simulink model "os_polyphase_synth_4096c"  (R2021a) (continued)
== system os_polyphase_synth_4096c/pfb_fir/pol1_in1_tap82 == (continued)
      | PCIN>>17+(A+D)*(A+D)+C+CARRYIN
      | PCIN>>17+(A+D)*(A+D)+CARRYIN
      | PCIN>>17+(A+D)*(A+D)+P
      | PCIN>>17+(A+D)*(A+D)+P+CARRYIN
      | PCIN>>17+(A+D)*B
      | PCIN>>17+(A+D)*B+C
      | PCIN>>17+(A+D)*B+C+CARRYIN
      | PCIN>>17+(A+D)*B+CARRYIN
      | PCIN>>17+(A+D)*B+P
      | PCIN>>17+(A+D)*B+P+CARRYIN
      | PCIN>>17+(A+D)*BCIN
      | PCIN>>17+(A+D)*BCIN+C
      | PCIN>>17+(A+D)*BCIN+C+CARRYIN
      | PCIN>>17+(A+D)*BCIN+CARRYIN
      | PCIN>>17+(A+D)*BCIN+P
      | PCIN>>17+(A+D)*BCIN+P+CARRYIN
      | PCIN>>17+(A+D)+C
      | PCIN>>17+(A+D)+C+CARRYIN
      | PCIN>>17+(A+D)+CARRYIN
      | PCIN>>17+(A+D)+P
      | PCIN>>17+(A+D)+P+CARRYIN
      | PCIN>>17+(ACIN+D)
      | PCIN>>17+(ACIN+D)*(ACIN+D)
      | PCIN>>17+(ACIN+D)*(ACIN+D)+C
      | PCIN>>17+(ACIN+D)*(ACIN+D)+C+CARRYIN
      | PCIN>>17+(ACIN+D)*(ACIN+D)+CARRYIN
      | PCIN>>17+(ACIN+D)*(ACIN+D)+P
      | PCIN>>17+(ACIN+D)*(ACIN+D)+P+CARRYIN
      | PCIN>>17+(ACIN+D)*B
      | PCIN>>17+(ACIN+D)*B+C
      | PCIN>>17+(ACIN+D)*B+C+CARRYIN
      | PCIN>>17+(ACIN+D)*B+CARRYIN
      | PCIN>>17+(ACIN+D)*B+P
      | PCIN>>17+(ACIN+D)*B+P+CARRYIN
      | PCIN>>17+(ACIN+D)*BCIN
      | PCIN>>17+(ACIN+D)*BCIN+C
      | PCIN>>17+(ACIN+D)*BCIN+C+CARRYIN
      | PCIN>>17+(ACIN+D)*BCIN+CARRYIN
      | PCIN>>17+(ACIN+D)*BCIN+P
      | PCIN>>17+(ACIN+D)*BCIN+P+CARRYIN
      | PCIN>>17+(ACIN+D)+C
      | PCIN>>17+(ACIN+D)+C+CARRYIN
      | PCIN>>17+(ACIN+D)+CARRYIN
      | PCIN>>17+(ACIN+D)+P
      | PCIN>>17+(ACIN+D)+P+CARRYIN
      | PCIN>>17+(D+A)
      | PCIN>>17+(D+A)*(D+A)
      | PCIN>>17+(D+A)*(D+A)+C
      | PCIN>>17+(D+A)*(D+A)+C+CARRYIN
      | PCIN>>17+(D+A)*(D+A)+CARRYIN
      | PCIN>>17+(D+A)*(D+A)+P
      | PCIN>>17+(D+A)*(D+A)+P+CARRYIN
      | PCIN>>17+(D+A)*B
      | PCIN>>17+(D+A)*B+C
      | PCIN>>17+(D+A)*B+C+CARRYIN
      | PCIN>>17+(D+A)*B+CARRYIN
      | PCIN>>17+(D+A)*B+P
      | PCIN>>17+(D+A)*B+P+CARRYIN
      | PCIN>>17+(D+A)*BCIN
      | PCIN>>17+(D+A)*BCIN+C
      | PCIN>>17+(D+A)*BCIN+C+CARRYIN
      | PCIN>>17+(D+A)*BCIN+CARRYIN
      | PCIN>>17+(D+A)*BCIN+P
      | PCIN>>17+(D+A)*BCIN+P+CARRYIN
      | PCIN>>17+(D+A)+C
      | PCIN>>17+(D+A)+C+CARRYIN
      | PCIN>>17+(D+A)+CARRYIN
      | PCIN>>17+(D+A)+P
      | PCIN>>17+(D+A)+P+CARRYIN
      | PCIN>>17+(D+ACIN)
      | PCIN>>17+(D+ACIN)*(D+ACIN)
      | PCIN>>17+(D+ACIN)*(D+ACIN)+C
      | PCIN>>17+(D+ACIN)*(D+ACIN)+C+CARRYIN
      | PCIN>>17+(D+ACIN)*(D+ACIN)+CARRYIN
      | PCIN>>17+(D+ACIN)*(D+ACIN)+P
      | PCIN>>17+(D+ACIN)*(D+ACIN)+P+CARRYIN
      | PCIN>>17+(D+ACIN)*B
      | PCIN>>17+(D+ACIN)*B+C
      | PCIN>>17+(D+ACIN)*B+C+CARRYIN
      | PCIN>>17+(D+ACIN)*B+CARRYIN
      | PCIN>>17+(D+ACIN)*B+P
      | PCIN>>17+(D+ACIN)*B+P+CARRYIN
      | PCIN>>17+(D+ACIN)*BCIN
      | PCIN>>17+(D+ACIN)*BCIN+C
      | PCIN>>17+(D+ACIN)*BCIN+C+CARRYIN
      | PCIN>>17+(D+ACIN)*BCIN+CARRYIN
      | PCIN>>17+(D+ACIN)*BCIN+P
      | PCIN>>17+(D+ACIN)*BCIN+P+CARRYIN
      | PCIN>>17+(D+ACIN)+C
      | PCIN>>17+(D+ACIN)+C+CARRYIN
      | PCIN>>17+(D+ACIN)+CARRYIN
      | PCIN>>17+(D+ACIN)+P
      | PCIN>>17+(D+ACIN)+P+CARRYIN
      | PCIN>>17+(D-A)
      | PCIN>>17+(D-A)*(D-A)
      | PCIN>>17+(D-A)*(D-A)+C
      | PCIN>>17+(D-A)*(D-A)+C+CARRYIN
      | PCIN>>17+(D-A)*(D-A)+CARRYIN
      | PCIN>>17+(D-A)*(D-A)+P
      | PCIN>>17+(D-A)*(D-A)+P+CARRYIN
      | PCIN>>17+(D-A)*B
      | PCIN>>17+(D-A)*B+C
      | PCIN>>17+(D-A)*B+C+CARRYIN
      | PCIN>>17+(D-A)*B+CARRYIN
      | PCIN>>17+(D-A)*B+P
      | PCIN>>17+(D-A)*B+P+CARRYIN
      | PCIN>>17+(D-A)*BCIN
      | PCIN>>17+(D-A)*BCIN+C
      | PCIN>>17+(D-A)*BCIN+C+CARRYIN
      | PCIN>>17+(D-A)*BCIN+CARRYIN
      | PCIN>>17+(D-A)*BCIN+P
      | PCIN>>17+(D-A)*BCIN+P+CARRYIN
      | PCIN>>17+(D-A)+C
      | PCIN>>17+(D-A)+C+CARRYIN
      | PCIN>>17+(D-A)+CARRYIN
      | PCIN>>17+(D-A)+P
      | PCIN>>17+(D-A)+P+CARRYIN
      | PCIN>>17+(D-ACIN)
      | PCIN>>17+(D-ACIN)*(D-ACIN)
      | PCIN>>17+(D-ACIN)*(D-ACIN)+C
      | PCIN>>17+(D-ACIN)*(D-ACIN)+C+CARRYIN
      | PCIN>>17+(D-ACIN)*(D-ACIN)+CARRYIN
      | PCIN>>17+(D-ACIN)*(D-ACIN)+P
      | PCIN>>17+(D-ACIN)*(D-ACIN)+P+CARRYIN
      | PCIN>>17+(D-ACIN)*B
      | PCIN>>17+(D-ACIN)*B+C
      | PCIN>>17+(D-ACIN)*B+C+CARRYIN
      | PCIN>>17+(D-ACIN)*B+CARRYIN
      | PCIN>>17+(D-ACIN)*B+P
      | PCIN>>17+(D-ACIN)*B+P+CARRYIN
      | PCIN>>17+(D-ACIN)*BCIN
      | PCIN>>17+(D-ACIN)*BCIN+C
      | PCIN>>17+(D-ACIN)*BCIN+C+CARRYIN
      | PCIN>>17+(D-ACIN)*BCIN+CARRYIN
      | PCIN>>17+(D-ACIN)*BCIN+P
      | PCIN>>17+(D-ACIN)*BCIN+P+CARRYIN
      | PCIN>>17+(D-ACIN)+C
      | PCIN>>17+(D-ACIN)+C+CARRYIN
      | PCIN>>17+(D-ACIN)+CARRYIN
      | PCIN>>17+(D-ACIN)+P
      | PCIN>>17+(D-ACIN)+P+CARRYIN
      | PCIN>>17+A
      | PCIN>>17+A*A
      | PCIN>>17+A*A+C
      | PCIN>>17+A*A+C+CARRYIN
      | PCIN>>17+A*A+CARRYIN
      | PCIN>>17+A*A+P
      | PCIN>>17+A*A+P+CARRYIN
      | PCIN>>17+A*B
      | PCIN>>17+A*B+C
      | PCIN>>17+A*B+C+CARRYIN
      | PCIN>>17+A*B+CARRYIN
      | PCIN>>17+A*B+P
      | PCIN>>17+A*B+P+CARRYIN
      | PCIN>>17+A*BCIN
      | PCIN>>17+A*BCIN+C
      | PCIN>>17+A*BCIN+C+CARRYIN
      | PCIN>>17+A*BCIN+CARRYIN
      | PCIN>>17+A*BCIN+P
      | PCIN>>17+A*BCIN+P+CARRYIN
      | PCIN>>17+A+C
      | PCIN>>17+A+C+CARRYIN
      | PCIN>>17+A+CARRYIN
      | PCIN>>17+A+P
      | PCIN>>17+A+P+CARRYIN
      | PCIN>>17+ACIN
      | PCIN>>17+ACIN*ACIN
      | PCIN>>17+ACIN*ACIN+C
      | PCIN>>17+ACIN*ACIN+C+CARRYIN
      | PCIN>>17+ACIN*ACIN+CARRYIN
      | PCIN>>17+ACIN*ACIN+P
      | PCIN>>17+ACIN*ACIN+P+CARRYIN
      | PCIN>>17+ACIN*B
      | PCIN>>17+ACIN*B+C
      | PCIN>>17+ACIN*B+C+CARRYIN
      | PCIN>>17+ACIN*B+CARRYIN
      | PCIN>>17+ACIN*B+P
      | PCIN>>17+ACIN*B+P+CARRYIN
      | PCIN>>17+ACIN*BCIN
      | PCIN>>17+ACIN*BCIN+C
      | PCIN>>17+ACIN*BCIN+C+CARRYIN
      | PCIN>>17+ACIN*BCIN+CARRYIN
      | PCIN>>17+ACIN*BCIN+P
      | PCIN>>17+ACIN*BCIN+P+CARRYIN
      | PCIN>>17+ACIN+C
      | PCIN>>17+ACIN+C+CARRYIN
      | PCIN>>17+ACIN+CARRYIN
      | PCIN>>17+ACIN+P
      | PCIN>>17+ACIN+P+CARRYIN
      | PCIN>>17+B*(A+D)
      | PCIN>>17+B*(A+D)+C
      | PCIN>>17+B*(A+D)+C+CARRYIN
      | PCIN>>17+B*(A+D)+CARRYIN
      | PCIN>>17+B*(A+D)+P
      | PCIN>>17+B*(A+D)+P+CARRYIN
      | PCIN>>17+B*(ACIN+D)
      | PCIN>>17+B*(ACIN+D)+C
      | PCIN>>17+B*(ACIN+D)+C+CARRYIN
      | PCIN>>17+B*(ACIN+D)+CARRYIN
      | PCIN>>17+B*(ACIN+D)+P
      | PCIN>>17+B*(ACIN+D)+P+CARRYIN
      | PCIN>>17+B*(D+A)
      | PCIN>>17+B*(D+A)+C
      | PCIN>>17+B*(D+A)+C+CARRYIN
      | PCIN>>17+B*(D+A)+CARRYIN
      | PCIN>>17+B*(D+A)+P
      | PCIN>>17+B*(D+A)+P+CARRYIN
      | PCIN>>17+B*(D+ACIN)
      | PCIN>>17+B*(D+ACIN)+C
      | PCIN>>17+B*(D+ACIN)+C+CARRYIN
      | PCIN>>17+B*(D+ACIN)+CARRYIN
      | PCIN>>17+B*(D+ACIN)+P
      | PCIN>>17+B*(D+ACIN)+P+CARRYIN
      | PCIN>>17+B*(D-A)
      | PCIN>>17+B*(D-A)+C
      | PCIN>>17+B*(D-A)+C+CARRYIN
      | PCIN>>17+B*(D-A)+CARRYIN
      | PCIN>>17+B*(D-A)+P
      | PCIN>>17+B*(D-A)+P+CARRYIN
      | PCIN>>17+B*(D-ACIN)
      | PCIN>>17+B*(D-ACIN)+C
      | PCIN>>17+B*(D-ACIN)+C+CARRYIN
      | PCIN>>17+B*(D-ACIN)+CARRYIN
      | PCIN>>17+B*(D-ACIN)+P
      | PCIN>>17+B*(D-ACIN)+P+CARRYIN
      | PCIN>>17+B*A
      | PCIN>>17+B*A+C
      | PCIN>>17+B*A+C+CARRYIN
      | PCIN>>17+B*A+CARRYIN
      | PCIN>>17+B*A+P
      | PCIN>>17+B*A+P+CARRYIN
      | PCIN>>17+B*ACIN
      | PCIN>>17+B*ACIN+C
      | PCIN>>17+B*ACIN+C+CARRYIN
      | PCIN>>17+B*ACIN+CARRYIN
      | PCIN>>17+B*ACIN+P
      | PCIN>>17+B*ACIN+P+CARRYIN
      | PCIN>>17+B*D
      | PCIN>>17+B*D+C
      | PCIN>>17+B*D+C+CARRYIN
      | PCIN>>17+B*D+CARRYIN
      | PCIN>>17+B*D+P
      | PCIN>>17+B*D+P+CARRYIN
      | PCIN>>17+BCIN*(A+D)
      | PCIN>>17+BCIN*(A+D)+C
      | PCIN>>17+BCIN*(A+D)+C+CARRYIN
      | PCIN>>17+BCIN*(A+D)+CARRYIN
      | PCIN>>17+BCIN*(A+D)+P
      | PCIN>>17+BCIN*(A+D)+P+CARRYIN
      | PCIN>>17+BCIN*(ACIN+D)
      | PCIN>>17+BCIN*(ACIN+D)+C
      | PCIN>>17+BCIN*(ACIN+D)+C+CARRYIN
      | PCIN>>17+BCIN*(ACIN+D)+CARRYIN
      | PCIN>>17+BCIN*(ACIN+D)+P
      | PCIN>>17+BCIN*(ACIN+D)+P+CARRYIN
      | PCIN>>17+BCIN*(D+A)
      | PCIN>>17+BCIN*(D+A)+C
      | PCIN>>17+BCIN*(D+A)+C+CARRYIN
      | PCIN>>17+BCIN*(D+A)+CARRYIN
      | PCIN>>17+BCIN*(D+A)+P
      | PCIN>>17+BCIN*(D+A)+P+CARRYIN
      | PCIN>>17+BCIN*(D+ACIN)
      | PCIN>>17+BCIN*(D+ACIN)+C
      | PCIN>>17+BCIN*(D+ACIN)+C+CARRYIN
      | PCIN>>17+BCIN*(D+ACIN)+CARRYIN
      | PCIN>>17+BCIN*(D+ACIN)+P
      | PCIN>>17+BCIN*(D+ACIN)+P+CARRYIN
      | PCIN>>17+BCIN*(D-A)
      | PCIN>>17+BCIN*(D-A)+C
      | PCIN>>17+BCIN*(D-A)+C+CARRYIN
      | PCIN>>17+BCIN*(D-A)+CARRYIN
      | PCIN>>17+BCIN*(D-A)+P
      | PCIN>>17+BCIN*(D-A)+P+CARRYIN
      | PCIN>>17+BCIN*(D-ACIN)
      | PCIN>>17+BCIN*(D-ACIN)+C
      | PCIN>>17+BCIN*(D-ACIN)+C+CARRYIN
      | PCIN>>17+BCIN*(D-ACIN)+CARRYIN
      | PCIN>>17+BCIN*(D-ACIN)+P
      | PCIN>>17+BCIN*(D-ACIN)+P+CARRYIN
      | PCIN>>17+BCIN*A
      | PCIN>>17+BCIN*A+C
      | PCIN>>17+BCIN*A+C+CARRYIN
      | PCIN>>17+BCIN*A+CARRYIN
      | PCIN>>17+BCIN*A+P
      | PCIN>>17+BCIN*A+P+CARRYIN
      | PCIN>>17+BCIN*ACIN
      | PCIN>>17+BCIN*ACIN+C
      | PCIN>>17+BCIN*ACIN+C+CARRYIN
      | PCIN>>17+BCIN*ACIN+CARRYIN
      | PCIN>>17+BCIN*ACIN+P
      | PCIN>>17+BCIN*ACIN+P+CARRYIN
      | PCIN>>17+BCIN*D
      | PCIN>>17+BCIN*D+C
      | PCIN>>17+BCIN*D+C+CARRYIN
      | PCIN>>17+BCIN*D+CARRYIN
      | PCIN>>17+BCIN*D+P
      | PCIN>>17+BCIN*D+P+CARRYIN
      | PCIN>>17+C
      | PCIN>>17+C+(A+D)
      | PCIN>>17+C+(A+D)*(A+D)
      | PCIN>>17+C+(A+D)*(A+D)+CARRYIN
      | PCIN>>17+C+(A+D)*B
      | PCIN>>17+C+(A+D)*B+CARRYIN
      | PCIN>>17+C+(A+D)*BCIN
      | PCIN>>17+C+(A+D)*BCIN+CARRYIN
      | PCIN>>17+C+(A+D)+CARRYIN
      | PCIN>>17+C+(ACIN+D)
      | PCIN>>17+C+(ACIN+D)*(ACIN+D)
      | PCIN>>17+C+(ACIN+D)*(ACIN+D)+CARRYIN
      | PCIN>>17+C+(ACIN+D)*B
      | PCIN>>17+C+(ACIN+D)*B+CARRYIN
      | PCIN>>17+C+(ACIN+D)*BCIN
      | PCIN>>17+C+(ACIN+D)*BCIN+CARRYIN
      | PCIN>>17+C+(ACIN+D)+CARRYIN
      | PCIN>>17+C+(D+A)
      | PCIN>>17+C+(D+A)*(D+A)
      | PCIN>>17+C+(D+A)*(D+A)+CARRYIN
      | PCIN>>17+C+(D+A)*B
      | PCIN>>17+C+(D+A)*B+CARRYIN
      | PCIN>>17+C+(D+A)*BCIN
      | PCIN>>17+C+(D+A)*BCIN+CARRYIN
      | PCIN>>17+C+(D+A)+CARRYIN
      | PCIN>>17+C+(D+ACIN)
      | PCIN>>17+C+(D+ACIN)*(D+ACIN)
      | PCIN>>17+C+(D+ACIN)*(D+ACIN)+CARRYIN
      | PCIN>>17+C+(D+ACIN)*B
      | PCIN>>17+C+(D+ACIN)*B+CARRYIN
      | PCIN>>17+C+(D+ACIN)*BCIN
      | PCIN>>17+C+(D+ACIN)*BCIN+CARRYIN
      | PCIN>>17+C+(D+ACIN)+CARRYIN
      | PCIN>>17+C+(D-A)
      | PCIN>>17+C+(D-A)*(D-A)
      | PCIN>>17+C+(D-A)*(D-A)+CARRYIN
      | PCIN>>17+C+(D-A)*B
      | PCIN>>17+C+(D-A)*B+CARRYIN
      | PCIN>>17+C+(D-A)*BCIN
      | PCIN>>17+C+(D-A)*BCIN+CARRYIN
      | PCIN>>17+C+(D-A)+CARRYIN
      | PCIN>>17+C+(D-ACIN)
      | PCIN>>17+C+(D-ACIN)*(D-ACIN)
      | PCIN>>17+C+(D-ACIN)*(D-ACIN)+CARRYIN
      | PCIN>>17+C+(D-ACIN)*B
      | PCIN>>17+C+(D-ACIN)*B+CARRYIN
      | PCIN>>17+C+(D-ACIN)*BCIN
      | PCIN>>17+C+(D-ACIN)*BCIN+CARRYIN
      | PCIN>>17+C+(D-ACIN)+CARRYIN
      | PCIN>>17+C+A
      | PCIN>>17+C+A*A
      | PCIN>>17+C+A*A+CARRYIN
      | PCIN>>17+C+A*B
      | PCIN>>17+C+A*B+CARRYIN
      | PCIN>>17+C+A*BCIN
      | PCIN>>17+C+A*BCIN+CARRYIN
      | PCIN>>17+C+A+CARRYIN
      | PCIN>>17+C+ACIN
      | PCIN>>17+C+ACIN*ACIN
      | PCIN>>17+C+ACIN*ACIN+CARRYIN
      | PCIN>>17+C+ACIN*B
      | PCIN>>17+C+ACIN*B+CARRYIN
      | PCIN>>17+C+ACIN*BCIN
      | PCIN>>17+C+ACIN*BCIN+CARRYIN
      | PCIN>>17+C+ACIN+CARRYIN
      | PCIN>>17+C+B*(A+D)
      | PCIN>>17+C+B*(A+D)+CARRYIN
      | PCIN>>17+C+B*(ACIN+D)
      | PCIN>>17+C+B*(ACIN+D)+CARRYIN
      | PCIN>>17+C+B*(D+A)
      | PCIN>>17+C+B*(D+A)+CARRYIN
      | PCIN>>17+C+B*(D+ACIN)
      | PCIN>>17+C+B*(D+ACIN)+CARRYIN
      | PCIN>>17+C+B*(D-A)
      | PCIN>>17+C+B*(D-A)+CARRYIN
      | PCIN>>17+C+B*(D-ACIN)
      | PCIN>>17+C+B*(D-ACIN)+CARRYIN
      | PCIN>>17+C+B*A
      | PCIN>>17+C+B*A+CARRYIN
      | PCIN>>17+C+B*ACIN
      | PCIN>>17+C+B*ACIN+CARRYIN
      | PCIN>>17+C+B*D
      | PCIN>>17+C+B*D+CARRYIN
      | PCIN>>17+C+BCIN*(A+D)
      | PCIN>>17+C+BCIN*(A+D)+CARRYIN
      | PCIN>>17+C+BCIN*(ACIN+D)
      | PCIN>>17+C+BCIN*(ACIN+D)+CARRYIN
      | PCIN>>17+C+BCIN*(D+A)
      | PCIN>>17+C+BCIN*(D+A)+CARRYIN
      | PCIN>>17+C+BCIN*(D+ACIN)
      | PCIN>>17+C+BCIN*(D+ACIN)+CARRYIN
      | PCIN>>17+C+BCIN*(D-A)
      | PCIN>>17+C+BCIN*(D-A)+CARRYIN
      | PCIN>>17+C+BCIN*(D-ACIN)
      | PCIN>>17+C+BCIN*(D-ACIN)+CARRYIN
      | PCIN>>17+C+BCIN*A
      | PCIN>>17+C+BCIN*A+CARRYIN
      | PCIN>>17+C+BCIN*ACIN
      | PCIN>>17+C+BCIN*ACIN+CARRYIN
      | PCIN>>17+C+BCIN*D
      | PCIN>>17+C+BCIN*D+CARRYIN
      | PCIN>>17+C+C
      | PCIN>>17+C+C+CARRYIN
      | PCIN>>17+C+C+CONCAT
      | PCIN>>17+C+C+CONCAT+CARRYIN
      | PCIN>>17+C+C+P
      | PCIN>>17+C+C+P+CARRYIN
      | PCIN>>17+C+CARRYIN
      | PCIN>>17+C+CONCAT
      | PCIN>>17+C+CONCAT+C
      | PCIN>>17+C+CONCAT+C+CARRYIN
      | PCIN>>17+C+CONCAT+CARRYIN
      | PCIN>>17+C+CONCAT+P
      | PCIN>>17+C+CONCAT+P+CARRYIN
      | PCIN>>17+C+D
      | PCIN>>17+C+D*B
      | PCIN>>17+C+D*B+CARRYIN
      | PCIN>>17+C+D*BCIN
      | PCIN>>17+C+D*BCIN+CARRYIN
      | PCIN>>17+C+D*D
      | PCIN>>17+C+D*D+CARRYIN
      | PCIN>>17+C+D+CARRYIN
      | PCIN>>17+C+P
      | PCIN>>17+C+P+C
      | PCIN>>17+C+P+C+CARRYIN
      | PCIN>>17+C+P+CARRYIN
      | PCIN>>17+C+P+CONCAT
      | PCIN>>17+C+P+CONCAT+CARRYIN
      | PCIN>>17+C+P+P
      | PCIN>>17+C+P+P+CARRYIN
      | PCIN>>17+CARRYIN
      | PCIN>>17+CONCAT
      | PCIN>>17+CONCAT+C
      | PCIN>>17+CONCAT+C+C
      | PCIN>>17+CONCAT+C+C+CARRYIN
      | PCIN>>17+CONCAT+C+CARRYIN
      | PCIN>>17+CONCAT+C+P
      | PCIN>>17+CONCAT+C+P+CARRYIN
      | PCIN>>17+CONCAT+CARRYIN
      | PCIN>>17+CONCAT+P
      | PCIN>>17+CONCAT+P+C
      | PCIN>>17+CONCAT+P+C+CARRYIN
      | PCIN>>17+CONCAT+P+CARRYIN
      | PCIN>>17+D
      | PCIN>>17+D*B
      | PCIN>>17+D*B+C
      | PCIN>>17+D*B+C+CARRYIN
      | PCIN>>17+D*B+CARRYIN
      | PCIN>>17+D*B+P
      | PCIN>>17+D*B+P+CARRYIN
      | PCIN>>17+D*BCIN
      | PCIN>>17+D*BCIN+C
      | PCIN>>17+D*BCIN+C+CARRYIN
      | PCIN>>17+D*BCIN+CARRYIN
      | PCIN>>17+D*BCIN+P
      | PCIN>>17+D*BCIN+P+CARRYIN
      | PCIN>>17+D*D
      | PCIN>>17+D*D+C
      | PCIN>>17+D*D+C+CARRYIN
      | PCIN>>17+D*D+CARRYIN
      | PCIN>>17+D*D+P
      | PCIN>>17+D*D+P+CARRYIN
      | PCIN>>17+D+C
      | PCIN>>17+D+C+CARRYIN
      | PCIN>>17+D+CARRYIN
      | PCIN>>17+D+P
      | PCIN>>17+D+P+CARRYIN
      | PCIN>>17+P
      | PCIN>>17+P+(A+D)
      | PCIN>>17+P+(A+D)*(A+D)
      | PCIN>>17+P+(A+D)*(A+D)+CARRYIN
      | PCIN>>17+P+(A+D)*B
      | PCIN>>17+P+(A+D)*B+CARRYIN
      | PCIN>>17+P+(A+D)*BCIN
      | PCIN>>17+P+(A+D)*BCIN+CARRYIN
      | PCIN>>17+P+(A+D)+CARRYIN
      | PCIN>>17+P+(ACIN+D)
      | PCIN>>17+P+(ACIN+D)*(ACIN+D)
      | PCIN>>17+P+(ACIN+D)*(ACIN+D)+CARRYIN
      | PCIN>>17+P+(ACIN+D)*B
      | PCIN>>17+P+(ACIN+D)*B+CARRYIN
      | PCIN>>17+P+(ACIN+D)*BCIN
      | PCIN>>17+P+(ACIN+D)*BCIN+CARRYIN
      | PCIN>>17+P+(ACIN+D)+CARRYIN
      | PCIN>>17+P+(D+A)
      | PCIN>>17+P+(D+A)*(D+A)
      | PCIN>>17+P+(D+A)*(D+A)+CARRYIN
      | PCIN>>17+P+(D+A)*B
      | PCIN>>17+P+(D+A)*B+CARRYIN
      | PCIN>>17+P+(D+A)*BCIN
      | PCIN>>17+P+(D+A)*BCIN+CARRYIN
      | PCIN>>17+P+(D+A)+CARRYIN
      | PCIN>>17+P+(D+ACIN)
      | PCIN>>17+P+(D+ACIN)*(D+ACIN)
      | PCIN>>17+P+(D+ACIN)*(D+ACIN)+CARRYIN
      | PCIN>>17+P+(D+ACIN)*B
      | PCIN>>17+P+(D+ACIN)*B+CARRYIN
      | PCIN>>17+P+(D+ACIN)*BCIN
      | PCIN>>17+P+(D+ACIN)*BCIN+CARRYIN
      | PCIN>>17+P+(D+ACIN)+CARRYIN
      | PCIN>>17+P+(D-A)
      | PCIN>>17+P+(D-A)*(D-A)
      | PCIN>>17+P+(D-A)*(D-A)+CARRYIN
      | PCIN>>17+P+(D-A)*B
      | PCIN>>17+P+(D-A)*B+CARRYIN
      | PCIN>>17+P+(D-A)*BCIN
      | PCIN>>17+P+(D-A)*BCIN+CARRYIN
      | PCIN>>17+P+(D-A)+CARRYIN
      | PCIN>>17+P+(D-ACIN)
      | PCIN>>17+P+(D-ACIN)*(D-ACIN)
      | PCIN>>17+P+(D-ACIN)*(D-ACIN)+CARRYIN
      | PCIN>>17+P+(D-ACIN)*B
      | PCIN>>17+P+(D-ACIN)*B+CARRYIN
      | PCIN>>17+P+(D-ACIN)*BCIN
      | PCIN>>17+P+(D-ACIN)*BCIN+CARRYIN
      | PCIN>>17+P+(D-ACIN)+CARRYIN
      | PCIN>>17+P+A
      | PCIN>>17+P+A*A
      | PCIN>>17+P+A*A+CARRYIN
      | PCIN>>17+P+A*B
      | PCIN>>17+P+A*B+CARRYIN
      | PCIN>>17+P+A*BCIN
      | PCIN>>17+P+A*BCIN+CARRYIN
      | PCIN>>17+P+A+CARRYIN
      | PCIN>>17+P+ACIN
      | PCIN>>17+P+ACIN*ACIN
      | PCIN>>17+P+ACIN*ACIN+CARRYIN
      | PCIN>>17+P+ACIN*B
      | PCIN>>17+P+ACIN*B+CARRYIN
      | PCIN>>17+P+ACIN*BCIN
      | PCIN>>17+P+ACIN*BCIN+CARRYIN
      | PCIN>>17+P+ACIN+CARRYIN
      | PCIN>>17+P+B*(A+D)
      | PCIN>>17+P+B*(A+D)+CARRYIN
      | PCIN>>17+P+B*(ACIN+D)
      | PCIN>>17+P+B*(ACIN+D)+CARRYIN
      | PCIN>>17+P+B*(D+A)
      | PCIN>>17+P+B*(D+A)+CARRYIN
      | PCIN>>17+P+B*(D+ACIN)
      | PCIN>>17+P+B*(D+ACIN)+CARRYIN
      | PCIN>>17+P+B*(D-A)
      | PCIN>>17+P+B*(D-A)+CARRYIN
      | PCIN>>17+P+B*(D-ACIN)
      | PCIN>>17+P+B*(D-ACIN)+CARRYIN
      | PCIN>>17+P+B*A
      | PCIN>>17+P+B*A+CARRYIN
      | PCIN>>17+P+B*ACIN
      | PCIN>>17+P+B*ACIN+CARRYIN
      | PCIN>>17+P+B*D
      | PCIN>>17+P+B*D+CARRYIN
      | PCIN>>17+P+BCIN*(A+D)
      | PCIN>>17+P+BCIN*(A+D)+CARRYIN
      | PCIN>>17+P+BCIN*(ACIN+D)
      | PCIN>>17+P+BCIN*(ACIN+D)+CARRYIN
      | PCIN>>17+P+BCIN*(D+A)
      | PCIN>>17+P+BCIN*(D+A)+CARRYIN
      | PCIN>>17+P+BCIN*(D+ACIN)
      | PCIN>>17+P+BCIN*(D+ACIN)+CARRYIN
      | PCIN>>17+P+BCIN*(D-A)
      | PCIN>>17+P+BCIN*(D-A)+CARRYIN
      | PCIN>>17+P+BCIN*(D-ACIN)
      | PCIN>>17+P+BCIN*(D-ACIN)+CARRYIN
      | PCIN>>17+P+BCIN*A
      | PCIN>>17+P+BCIN*A+CARRYIN
      | PCIN>>17+P+BCIN*ACIN
      | PCIN>>17+P+BCIN*ACIN+CARRYIN
      | PCIN>>17+P+BCIN*D
      | PCIN>>17+P+BCIN*D+CARRYIN
      | PCIN>>17+P+C
      | PCIN>>17+P+C+C
      | PCIN>>17+P+C+C+CARRYIN
      | PCIN>>17+P+C+CARRYIN
      | PCIN>>17+P+C+CONCAT
      | PCIN>>17+P+C+CONCAT+CARRYIN
      | PCIN>>17+P+C+P
      | PCIN>>17+P+C+P+CARRYIN
      | PCIN>>17+P+CARRYIN
      | PCIN>>17+P+CONCAT
      | PCIN>>17+P+CONCAT+C
      | PCIN>>17+P+CONCAT+C+CARRYIN
      | PCIN>>17+P+CONCAT+CARRYIN
      | PCIN>>17+P+D
      | PCIN>>17+P+D*B
      | PCIN>>17+P+D*B+CARRYIN
      | PCIN>>17+P+D*BCIN
      | PCIN>>17+P+D*BCIN+CARRYIN
      | PCIN>>17+P+D*D
      | PCIN>>17+P+D*D+CARRYIN
      | PCIN>>17+P+D+CARRYIN
      | PCIN>>17+P+P
      | PCIN>>17+P+P+C
      | PCIN>>17+P+P+C+CARRYIN
      | PCIN>>17+P+P+CARRYIN
      | PCIN>>17-((A+D))
      | PCIN>>17-((A+D)*(A+D))
      | PCIN>>17-((A+D)*(A+D)+C)
      | PCIN>>17-((A+D)*(A+D)+C+CARRYIN)
      | PCIN>>17-((A+D)*(A+D)+CARRYIN)
      | PCIN>>17-((A+D)*(A+D)+P)
      | PCIN>>17-((A+D)*(A+D)+P+CARRYIN)
      | PCIN>>17-((A+D)*B)
      | PCIN>>17-((A+D)*B+C)
      | PCIN>>17-((A+D)*B+C+CARRYIN)
      | PCIN>>17-((A+D)*B+CARRYIN)
      | PCIN>>17-((A+D)*B+P)
      | PCIN>>17-((A+D)*B+P+CARRYIN)
      | PCIN>>17-((A+D)*BCIN)
      | PCIN>>17-((A+D)*BCIN+C)
      | PCIN>>17-((A+D)*BCIN+C+CARRYIN)
      | PCIN>>17-((A+D)*BCIN+CARRYIN)
      | PCIN>>17-((A+D)*BCIN+P)
      | PCIN>>17-((A+D)*BCIN+P+CARRYIN)
      | PCIN>>17-((A+D)+C)
      | PCIN>>17-((A+D)+C+CARRYIN)
      | PCIN>>17-((A+D)+CARRYIN)
      | PCIN>>17-((A+D)+P)
      | PCIN>>17-((A+D)+P+CARRYIN)
      | PCIN>>17-((ACIN+D))
      | PCIN>>17-((ACIN+D)*(ACIN+D))
      | PCIN>>17-((ACIN+D)*(ACIN+D)+C)
      | PCIN>>17-((ACIN+D)*(ACIN+D)+C+CARRYIN)
      | PCIN>>17-((ACIN+D)*(ACIN+D)+CARRYIN)
      | PCIN>>17-((ACIN+D)*(ACIN+D)+P)
      | PCIN>>17-((ACIN+D)*(ACIN+D)+P+CARRYIN)
      | PCIN>>17-((ACIN+D)*B)
      | PCIN>>17-((ACIN+D)*B+C)
      | PCIN>>17-((ACIN+D)*B+C+CARRYIN)
      | PCIN>>17-((ACIN+D)*B+CARRYIN)
      | PCIN>>17-((ACIN+D)*B+P)
      | PCIN>>17-((ACIN+D)*B+P+CARRYIN)
      | PCIN>>17-((ACIN+D)*BCIN)
      | PCIN>>17-((ACIN+D)*BCIN+C)
      | PCIN>>17-((ACIN+D)*BCIN+C+CARRYIN)
      | PCIN>>17-((ACIN+D)*BCIN+CARRYIN)
      | PCIN>>17-((ACIN+D)*BCIN+P)
      | PCIN>>17-((ACIN+D)*BCIN+P+CARRYIN)
      | PCIN>>17-((ACIN+D)+C)
      | PCIN>>17-((ACIN+D)+C+CARRYIN)
      | PCIN>>17-((ACIN+D)+CARRYIN)
      | PCIN>>17-((ACIN+D)+P)
      | PCIN>>17-((ACIN+D)+P+CARRYIN)
      | PCIN>>17-((D+A))
      | PCIN>>17-((D+A)*(D+A))
      | PCIN>>17-((D+A)*(D+A)+C)
      | PCIN>>17-((D+A)*(D+A)+C+CARRYIN)
      | PCIN>>17-((D+A)*(D+A)+CARRYIN)
      | PCIN>>17-((D+A)*(D+A)+P)
      | PCIN>>17-((D+A)*(D+A)+P+CARRYIN)
      | PCIN>>17-((D+A)*B)
      | PCIN>>17-((D+A)*B+C)
      | PCIN>>17-((D+A)*B+C+CARRYIN)
      | PCIN>>17-((D+A)*B+CARRYIN)
      | PCIN>>17-((D+A)*B+P)
      | PCIN>>17-((D+A)*B+P+CARRYIN)
      | PCIN>>17-((D+A)*BCIN)
      | PCIN>>17-((D+A)*BCIN+C)
      | PCIN>>17-((D+A)*BCIN+C+CARRYIN)
      | PCIN>>17-((D+A)*BCIN+CARRYIN)
      | PCIN>>17-((D+A)*BCIN+P)
      | PCIN>>17-((D+A)*BCIN+P+CARRYIN)
      | PCIN>>17-((D+A)+C)
      | PCIN>>17-((D+A)+C+CARRYIN)
      | PCIN>>17-((D+A)+CARRYIN)
      | PCIN>>17-((D+A)+P)
      | PCIN>>17-((D+A)+P+CARRYIN)
      | PCIN>>17-((D+ACIN))
      | PCIN>>17-((D+ACIN)*(D+ACIN))
      | PCIN>>17-((D+ACIN)*(D+ACIN)+C)
      | PCIN>>17-((D+ACIN)*(D+ACIN)+C+CARRYIN)
      | PCIN>>17-((D+ACIN)*(D+ACIN)+CARRYIN)
      | PCIN>>17-((D+ACIN)*(D+ACIN)+P)
      | PCIN>>17-((D+ACIN)*(D+ACIN)+P+CARRYIN)
      | PCIN>>17-((D+ACIN)*B)
      | PCIN>>17-((D+ACIN)*B+C)
      | PCIN>>17-((D+ACIN)*B+C+CARRYIN)
      | PCIN>>17-((D+ACIN)*B+CARRYIN)
      | PCIN>>17-((D+ACIN)*B+P)
      | PCIN>>17-((D+ACIN)*B+P+CARRYIN)
      | PCIN>>17-((D+ACIN)*BCIN)
      | PCIN>>17-((D+ACIN)*BCIN+C)
      | PCIN>>17-((D+ACIN)*BCIN+C+CARRYIN)
      | PCIN>>17-((D+ACIN)*BCIN+CARRYIN)
      | PCIN>>17-((D+ACIN)*BCIN+P)
      | PCIN>>17-((D+ACIN)*BCIN+P+CARRYIN)
      | PCIN>>17-((D+ACIN)+C)
      | PCIN>>17-((D+ACIN)+C+CARRYIN)
      | PCIN>>17-((D+ACIN)+CARRYIN)
      | PCIN>>17-((D+ACIN)+P)
      | PCIN>>17-((D+ACIN)+P+CARRYIN)
      | PCIN>>17-((D-A))
      | PCIN>>17-((D-A)*(D-A))
      | PCIN>>17-((D-A)*(D-A)+C)
      | PCIN>>17-((D-A)*(D-A)+C+CARRYIN)
      | PCIN>>17-((D-A)*(D-A)+CARRYIN)
      | PCIN>>17-((D-A)*(D-A)+P)
      | PCIN>>17-((D-A)*(D-A)+P+CARRYIN)
      | PCIN>>17-((D-A)*B)
      | PCIN>>17-((D-A)*B+C)
      | PCIN>>17-((D-A)*B+C+CARRYIN)
      | PCIN>>17-((D-A)*B+CARRYIN)
      | PCIN>>17-((D-A)*B+P)
      | PCIN>>17-((D-A)*B+P+CARRYIN)
      | PCIN>>17-((D-A)*BCIN)
      | PCIN>>17-((D-A)*BCIN+C)
      | PCIN>>17-((D-A)*BCIN+C+CARRYIN)
      | PCIN>>17-((D-A)*BCIN+CARRYIN)
      | PCIN>>17-((D-A)*BCIN+P)
      | PCIN>>17-((D-A)*BCIN+P+CARRYIN)
      | PCIN>>17-((D-A)+C)
      | PCIN>>17-((D-A)+C+CARRYIN)
      | PCIN>>17-((D-A)+CARRYIN)
      | PCIN>>17-((D-A)+P)
      | PCIN>>17-((D-A)+P+CARRYIN)
      | PCIN>>17-((D-ACIN))
      | PCIN>>17-((D-ACIN)*(D-ACIN))
      | PCIN>>17-((D-ACIN)*(D-ACIN)+C)
      | PCIN>>17-((D-ACIN)*(D-ACIN)+C+CARRYIN)
      | PCIN>>17-((D-ACIN)*(D-ACIN)+CARRYIN)
      | PCIN>>17-((D-ACIN)*(D-ACIN)+P)
      | PCIN>>17-((D-ACIN)*(D-ACIN)+P+CARRYIN)
      | PCIN>>17-((D-ACIN)*B)
      | PCIN>>17-((D-ACIN)*B+C)
      | PCIN>>17-((D-ACIN)*B+C+CARRYIN)
      | PCIN>>17-((D-ACIN)*B+CARRYIN)
      | PCIN>>17-((D-ACIN)*B+P)
      | PCIN>>17-((D-ACIN)*B+P+CARRYIN)
      | PCIN>>17-((D-ACIN)*BCIN)
      | PCIN>>17-((D-ACIN)*BCIN+C)
      | PCIN>>17-((D-ACIN)*BCIN+C+CARRYIN)
      | PCIN>>17-((D-ACIN)*BCIN+CARRYIN)
      | PCIN>>17-((D-ACIN)*BCIN+P)
      | PCIN>>17-((D-ACIN)*BCIN+P+CARRYIN)
      | PCIN>>17-((D-ACIN)+C)
      | PCIN>>17-((D-ACIN)+C+CARRYIN)
      | PCIN>>17-((D-ACIN)+CARRYIN)
      | PCIN>>17-((D-ACIN)+P)
      | PCIN>>17-((D-ACIN)+P+CARRYIN)
      | PCIN>>17-(A)
      | PCIN>>17-(A*A)
      | PCIN>>17-(A*A+C)
      | PCIN>>17-(A*A+C+CARRYIN)
      | PCIN>>17-(A*A+CARRYIN)
      | PCIN>>17-(A*A+P)
      | PCIN>>17-(A*A+P+CARRYIN)
      | PCIN>>17-(A*B)
      | PCIN>>17-(A*B+C)
      | PCIN>>17-(A*B+C+CARRYIN)
      | PCIN>>17-(A*B+CARRYIN)
      | PCIN>>17-(A*B+P)
      | PCIN>>17-(A*B+P+CARRYIN)
      | PCIN>>17-(A*BCIN)
      | PCIN>>17-(A*BCIN+C)
      | PCIN>>17-(A*BCIN+C+CARRYIN)
      | PCIN>>17-(A*BCIN+CARRYIN)
      | PCIN>>17-(A*BCIN+P)
      | PCIN>>17-(A*BCIN+P+CARRYIN)
      | PCIN>>17-(A+C)
      | PCIN>>17-(A+C+CARRYIN)
      | PCIN>>17-(A+CARRYIN)
      | PCIN>>17-(A+D)
      | PCIN>>17-(A+D)*(A+D)
      | PCIN>>17-(A+D)*(A+D)-C
      | PCIN>>17-(A+D)*(A+D)-C-CARRYIN
      | PCIN>>17-(A+D)*(A+D)-CARRYIN
      | PCIN>>17-(A+D)*(A+D)-P
      | PCIN>>17-(A+D)*(A+D)-P-CARRYIN
      | PCIN>>17-(A+D)*B
      | PCIN>>17-(A+D)*B-C
      | PCIN>>17-(A+D)*B-C-CARRYIN
      | PCIN>>17-(A+D)*B-CARRYIN
      | PCIN>>17-(A+D)*B-P
      | PCIN>>17-(A+D)*B-P-CARRYIN
      | PCIN>>17-(A+D)*BCIN
      | PCIN>>17-(A+D)*BCIN-C
      | PCIN>>17-(A+D)*BCIN-C-CARRYIN
      | PCIN>>17-(A+D)*BCIN-CARRYIN
      | PCIN>>17-(A+D)*BCIN-P
      | PCIN>>17-(A+D)*BCIN-P-CARRYIN
      | PCIN>>17-(A+D)-C
      | PCIN>>17-(A+D)-C-CARRYIN
      | PCIN>>17-(A+D)-CARRYIN
      | PCIN>>17-(A+D)-P
      | PCIN>>17-(A+D)-P-CARRYIN
      | PCIN>>17-(A+P)
      | PCIN>>17-(A+P+CARRYIN)
      | PCIN>>17-(ACIN)
      | PCIN>>17-(ACIN*ACIN)
      | PCIN>>17-(ACIN*ACIN+C)
      | PCIN>>17-(ACIN*ACIN+C+CARRYIN)
      | PCIN>>17-(ACIN*ACIN+CARRYIN)
      | PCIN>>17-(ACIN*ACIN+P)
      | PCIN>>17-(ACIN*ACIN+P+CARRYIN)
      | PCIN>>17-(ACIN*B)
      | PCIN>>17-(ACIN*B+C)
      | PCIN>>17-(ACIN*B+C+CARRYIN)
      | PCIN>>17-(ACIN*B+CARRYIN)
      | PCIN>>17-(ACIN*B+P)
      | PCIN>>17-(ACIN*B+P+CARRYIN)
      | PCIN>>17-(ACIN*BCIN)
      | PCIN>>17-(ACIN*BCIN+C)
      | PCIN>>17-(ACIN*BCIN+C+CARRYIN)
      | PCIN>>17-(ACIN*BCIN+CARRYIN)
      | PCIN>>17-(ACIN*BCIN+P)
      | PCIN>>17-(ACIN*BCIN+P+CARRYIN)
      | PCIN>>17-(ACIN+C)
      | PCIN>>17-(ACIN+C+CARRYIN)
      | PCIN>>17-(ACIN+CARRYIN)
      | PCIN>>17-(ACIN+D)
      | PCIN>>17-(ACIN+D)*(ACIN+D)
      | PCIN>>17-(ACIN+D)*(ACIN+D)-C
      | PCIN>>17-(ACIN+D)*(ACIN+D)-C-CARRYIN
      | PCIN>>17-(ACIN+D)*(ACIN+D)-CARRYIN
      | PCIN>>17-(ACIN+D)*(ACIN+D)-P
      | PCIN>>17-(ACIN+D)*(ACIN+D)-P-CARRYIN
      | PCIN>>17-(ACIN+D)*B
      | PCIN>>17-(ACIN+D)*B-C
      | PCIN>>17-(ACIN+D)*B-C-CARRYIN
      | PCIN>>17-(ACIN+D)*B-CARRYIN
      | PCIN>>17-(ACIN+D)*B-P
      | PCIN>>17-(ACIN+D)*B-P-CARRYIN
      | PCIN>>17-(ACIN+D)*BCIN
      | PCIN>>17-(ACIN+D)*BCIN-C
      | PCIN>>17-(ACIN+D)*BCIN-C-CARRYIN
      | PCIN>>17-(ACIN+D)*BCIN-CARRYIN
      | PCIN>>17-(ACIN+D)*BCIN-P
      | PCIN>>17-(ACIN+D)*BCIN-P-CARRYIN
      | PCIN>>17-(ACIN+D)-C
      | PCIN>>17-(ACIN+D)-C-CARRYIN
      | PCIN>>17-(ACIN+D)-CARRYIN
      | PCIN>>17-(ACIN+D)-P
      | PCIN>>17-(ACIN+D)-P-CARRYIN
      | PCIN>>17-(ACIN+P)
      | PCIN>>17-(ACIN+P+CARRYIN)
      | PCIN>>17-(B*(A+D))
      | PCIN>>17-(B*(A+D)+C)
      | PCIN>>17-(B*(A+D)+C+CARRYIN)
      | PCIN>>17-(B*(A+D)+CARRYIN)
      | PCIN>>17-(B*(A+D)+P)
      | PCIN>>17-(B*(A+D)+P+CARRYIN)
      | PCIN>>17-(B*(ACIN+D))
      | PCIN>>17-(B*(ACIN+D)+C)
      | PCIN>>17-(B*(ACIN+D)+C+CARRYIN)
      | PCIN>>17-(B*(ACIN+D)+CARRYIN)
      | PCIN>>17-(B*(ACIN+D)+P)
      | PCIN>>17-(B*(ACIN+D)+P+CARRYIN)
      | PCIN>>17-(B*(D+A))
      | PCIN>>17-(B*(D+A)+C)
      | PCIN>>17-(B*(D+A)+C+CARRYIN)
      | PCIN>>17-(B*(D+A)+CARRYIN)
      | PCIN>>17-(B*(D+A)+P)
      | PCIN>>17-(B*(D+A)+P+CARRYIN)
      | PCIN>>17-(B*(D+ACIN))
      | PCIN>>17-(B*(D+ACIN)+C)
      | PCIN>>17-(B*(D+ACIN)+C+CARRYIN)
      | PCIN>>17-(B*(D+ACIN)+CARRYIN)
      | PCIN>>17-(B*(D+ACIN)+P)
      | PCIN>>17-(B*(D+ACIN)+P+CARRYIN)
      | PCIN>>17-(B*(D-A))
      | PCIN>>17-(B*(D-A)+C)
      | PCIN>>17-(B*(D-A)+C+CARRYIN)
      | PCIN>>17-(B*(D-A)+CARRYIN)
      | PCIN>>17-(B*(D-A)+P)
      | PCIN>>17-(B*(D-A)+P+CARRYIN)
      | PCIN>>17-(B*(D-ACIN))
      | PCIN>>17-(B*(D-ACIN)+C)
      | PCIN>>17-(B*(D-ACIN)+C+CARRYIN)
      | PCIN>>17-(B*(D-ACIN)+CARRYIN)
      | PCIN>>17-(B*(D-ACIN)+P)
      | PCIN>>17-(B*(D-ACIN)+P+CARRYIN)
      | PCIN>>17-(B*A)
      | PCIN>>17-(B*A+C)
      | PCIN>>17-(B*A+C+CARRYIN)
      | PCIN>>17-(B*A+CARRYIN)
      | PCIN>>17-(B*A+P)
      | PCIN>>17-(B*A+P+CARRYIN)
      | PCIN>>17-(B*ACIN)
      | PCIN>>17-(B*ACIN+C)
      | PCIN>>17-(B*ACIN+C+CARRYIN)
      | PCIN>>17-(B*ACIN+CARRYIN)
      | PCIN>>17-(B*ACIN+P)
      | PCIN>>17-(B*ACIN+P+CARRYIN)
      | PCIN>>17-(B*D)
      | PCIN>>17-(B*D+C)
      | PCIN>>17-(B*D+C+CARRYIN)
      | PCIN>>17-(B*D+CARRYIN)
      | PCIN>>17-(B*D+P)
      | PCIN>>17-(B*D+P+CARRYIN)
      | PCIN>>17-(BCIN*(A+D))
      | PCIN>>17-(BCIN*(A+D)+C)
      | PCIN>>17-(BCIN*(A+D)+C+CARRYIN)
      | PCIN>>17-(BCIN*(A+D)+CARRYIN)
      | PCIN>>17-(BCIN*(A+D)+P)
      | PCIN>>17-(BCIN*(A+D)+P+CARRYIN)
      | PCIN>>17-(BCIN*(ACIN+D))
      | PCIN>>17-(BCIN*(ACIN+D)+C)
      | PCIN>>17-(BCIN*(ACIN+D)+C+CARRYIN)
      | PCIN>>17-(BCIN*(ACIN+D)+CARRYIN)
      | PCIN>>17-(BCIN*(ACIN+D)+P)
      | PCIN>>17-(BCIN*(ACIN+D)+P+CARRYIN)
      | PCIN>>17-(BCIN*(D+A))
      | PCIN>>17-(BCIN*(D+A)+C)
      | PCIN>>17-(BCIN*(D+A)+C+CARRYIN)
      | PCIN>>17-(BCIN*(D+A)+CARRYIN)
      | PCIN>>17-(BCIN*(D+A)+P)
      | PCIN>>17-(BCIN*(D+A)+P+CARRYIN)
      | PCIN>>17-(BCIN*(D+ACIN))
      | PCIN>>17-(BCIN*(D+ACIN)+C)
      | PCIN>>17-(BCIN*(D+ACIN)+C+CARRYIN)
      | PCIN>>17-(BCIN*(D+ACIN)+CARRYIN)
      | PCIN>>17-(BCIN*(D+ACIN)+P)
      | PCIN>>17-(BCIN*(D+ACIN)+P+CARRYIN)
      | PCIN>>17-(BCIN*(D-A))
      | PCIN>>17-(BCIN*(D-A)+C)
      | PCIN>>17-(BCIN*(D-A)+C+CARRYIN)
      | PCIN>>17-(BCIN*(D-A)+CARRYIN)
      | PCIN>>17-(BCIN*(D-A)+P)
      | PCIN>>17-(BCIN*(D-A)+P+CARRYIN)
      | PCIN>>17-(BCIN*(D-ACIN))
      | PCIN>>17-(BCIN*(D-ACIN)+C)
      | PCIN>>17-(BCIN*(D-ACIN)+C+CARRYIN)
      | PCIN>>17-(BCIN*(D-ACIN)+CARRYIN)
      | PCIN>>17-(BCIN*(D-ACIN)+P)
      | PCIN>>17-(BCIN*(D-ACIN)+P+CARRYIN)
      | PCIN>>17-(BCIN*A)
      | PCIN>>17-(BCIN*A+C)
      | PCIN>>17-(BCIN*A+C+CARRYIN)
      | PCIN>>17-(BCIN*A+CARRYIN)
      | PCIN>>17-(BCIN*A+P)
      | PCIN>>17-(BCIN*A+P+CARRYIN)
      | PCIN>>17-(BCIN*ACIN)
      | PCIN>>17-(BCIN*ACIN+C)
      | PCIN>>17-(BCIN*ACIN+C+CARRYIN)
      | PCIN>>17-(BCIN*ACIN+CARRYIN)
      | PCIN>>17-(BCIN*ACIN+P)
      | PCIN>>17-(BCIN*ACIN+P+CARRYIN)
      | PCIN>>17-(BCIN*D)
      | PCIN>>17-(BCIN*D+C)
      | PCIN>>17-(BCIN*D+C+CARRYIN)
      | PCIN>>17-(BCIN*D+CARRYIN)
      | PCIN>>17-(BCIN*D+P)
      | PCIN>>17-(BCIN*D+P+CARRYIN)
      | PCIN>>17-(C)
      | PCIN>>17-(C+(A+D))
      | PCIN>>17-(C+(A+D)*(A+D))
      | PCIN>>17-(C+(A+D)*(A+D)+CARRYIN)
      | PCIN>>17-(C+(A+D)*B)
      | PCIN>>17-(C+(A+D)*B+CARRYIN)
      | PCIN>>17-(C+(A+D)*BCIN)
      | PCIN>>17-(C+(A+D)*BCIN+CARRYIN)
      | PCIN>>17-(C+(A+D)+CARRYIN)
      | PCIN>>17-(C+(ACIN+D))
      | PCIN>>17-(C+(ACIN+D)*(ACIN+D))
      | PCIN>>17-(C+(ACIN+D)*(ACIN+D)+CARRYIN)
      | PCIN>>17-(C+(ACIN+D)*B)
      | PCIN>>17-(C+(ACIN+D)*B+CARRYIN)
      | PCIN>>17-(C+(ACIN+D)*BCIN)
      | PCIN>>17-(C+(ACIN+D)*BCIN+CARRYIN)
      | PCIN>>17-(C+(ACIN+D)+CARRYIN)
      | PCIN>>17-(C+(D+A))
      | PCIN>>17-(C+(D+A)*(D+A))
      | PCIN>>17-(C+(D+A)*(D+A)+CARRYIN)
      | PCIN>>17-(C+(D+A)*B)
      | PCIN>>17-(C+(D+A)*B+CARRYIN)
      | PCIN>>17-(C+(D+A)*BCIN)
      | PCIN>>17-(C+(D+A)*BCIN+CARRYIN)
      | PCIN>>17-(C+(D+A)+CARRYIN)
      | PCIN>>17-(C+(D+ACIN))
      | PCIN>>17-(C+(D+ACIN)*(D+ACIN))
      | PCIN>>17-(C+(D+ACIN)*(D+ACIN)+CARRYIN)
      | PCIN>>17-(C+(D+ACIN)*B)
      | PCIN>>17-(C+(D+ACIN)*B+CARRYIN)
      | PCIN>>17-(C+(D+ACIN)*BCIN)
      | PCIN>>17-(C+(D+ACIN)*BCIN+CARRYIN)
      | PCIN>>17-(C+(D+ACIN)+CARRYIN)
      | PCIN>>17-(C+(D-A))
      | PCIN>>17-(C+(D-A)*(D-A))
      | PCIN>>17-(C+(D-A)*(D-A)+CARRYIN)
      | PCIN>>17-(C+(D-A)*B)
      | PCIN>>17-(C+(D-A)*B+CARRYIN)
      | PCIN>>17-(C+(D-A)*BCIN)
      | PCIN>>17-(C+(D-A)*BCIN+CARRYIN)
      | PCIN>>17-(C+(D-A)+CARRYIN)
      | PCIN>>17-(C+(D-ACIN))
      | PCIN>>17-(C+(D-ACIN)*(D-ACIN))
      | PCIN>>17-(C+(D-ACIN)*(D-ACIN)+CARRYIN)
      | PCIN>>17-(C+(D-ACIN)*B)
      | PCIN>>17-(C+(D-ACIN)*B+CARRYIN)
      | PCIN>>17-(C+(D-ACIN)*BCIN)
      | PCIN>>17-(C+(D-ACIN)*BCIN+CARRYIN)
      | PCIN>>17-(C+(D-ACIN)+CARRYIN)
      | PCIN>>17-(C+A)
      | PCIN>>17-(C+A*A)
      | PCIN>>17-(C+A*A+CARRYIN)
      | PCIN>>17-(C+A*B)
      | PCIN>>17-(C+A*B+CARRYIN)
      | PCIN>>17-(C+A*BCIN)
      | PCIN>>17-(C+A*BCIN+CARRYIN)
      | PCIN>>17-(C+A+CARRYIN)
      | PCIN>>17-(C+ACIN)
      | PCIN>>17-(C+ACIN*ACIN)
      | PCIN>>17-(C+ACIN*ACIN+CARRYIN)
      | PCIN>>17-(C+ACIN*B)
      | PCIN>>17-(C+ACIN*B+CARRYIN)
      | PCIN>>17-(C+ACIN*BCIN)
      | PCIN>>17-(C+ACIN*BCIN+CARRYIN)
      | PCIN>>17-(C+ACIN+CARRYIN)
      | PCIN>>17-(C+B*(A+D))
      | PCIN>>17-(C+B*(A+D)+CARRYIN)
      | PCIN>>17-(C+B*(ACIN+D))
      | PCIN>>17-(C+B*(ACIN+D)+CARRYIN)
      | PCIN>>17-(C+B*(D+A))
      | PCIN>>17-(C+B*(D+A)+CARRYIN)
      | PCIN>>17-(C+B*(D+ACIN))
      | PCIN>>17-(C+B*(D+ACIN)+CARRYIN)
      | PCIN>>17-(C+B*(D-A))
      | PCIN>>17-(C+B*(D-A)+CARRYIN)
      | PCIN>>17-(C+B*(D-ACIN))
      | PCIN>>17-(C+B*(D-ACIN)+CARRYIN)
      | PCIN>>17-(C+B*A)
      | PCIN>>17-(C+B*A+CARRYIN)
      | PCIN>>17-(C+B*ACIN)
      | PCIN>>17-(C+B*ACIN+CARRYIN)
      | PCIN>>17-(C+B*D)
      | PCIN>>17-(C+B*D+CARRYIN)
      | PCIN>>17-(C+BCIN*(A+D))
      | PCIN>>17-(C+BCIN*(A+D)+CARRYIN)
      | PCIN>>17-(C+BCIN*(ACIN+D))
      | PCIN>>17-(C+BCIN*(ACIN+D)+CARRYIN)
      | PCIN>>17-(C+BCIN*(D+A))
      | PCIN>>17-(C+BCIN*(D+A)+CARRYIN)
      | PCIN>>17-(C+BCIN*(D+ACIN))
      | PCIN>>17-(C+BCIN*(D+ACIN)+CARRYIN)
      | PCIN>>17-(C+BCIN*(D-A))
      | PCIN>>17-(C+BCIN*(D-A)+CARRYIN)
      | PCIN>>17-(C+BCIN*(D-ACIN))
      | PCIN>>17-(C+BCIN*(D-ACIN)+CARRYIN)
      | PCIN>>17-(C+BCIN*A)
      | PCIN>>17-(C+BCIN*A+CARRYIN)
      | PCIN>>17-(C+BCIN*ACIN)
      | PCIN>>17-(C+BCIN*ACIN+CARRYIN)
      | PCIN>>17-(C+BCIN*D)
      | PCIN>>17-(C+BCIN*D+CARRYIN)
      | PCIN>>17-(C+C)
      | PCIN>>17-(C+C+CARRYIN)
      | PCIN>>17-(C+C+CONCAT)
      | PCIN>>17-(C+C+CONCAT+CARRYIN)
      | PCIN>>17-(C+C+P)
      | PCIN>>17-(C+C+P+CARRYIN)
      | PCIN>>17-(C+CARRYIN)
      | PCIN>>17-(C+CONCAT)
      | PCIN>>17-(C+CONCAT+C)
      | PCIN>>17-(C+CONCAT+C+CARRYIN)
      | PCIN>>17-(C+CONCAT+CARRYIN)
      | PCIN>>17-(C+CONCAT+P)
      | PCIN>>17-(C+CONCAT+P+CARRYIN)
      | PCIN>>17-(C+D)
      | PCIN>>17-(C+D*B)
      | PCIN>>17-(C+D*B+CARRYIN)
      | PCIN>>17-(C+D*BCIN)
      | PCIN>>17-(C+D*BCIN+CARRYIN)
      | PCIN>>17-(C+D*D)
      | PCIN>>17-(C+D*D+CARRYIN)
      | PCIN>>17-(C+D+CARRYIN)
      | PCIN>>17-(C+P)
      | PCIN>>17-(C+P+C)
      | PCIN>>17-(C+P+C+CARRYIN)
      | PCIN>>17-(C+P+CARRYIN)
      | PCIN>>17-(C+P+CONCAT)
      | PCIN>>17-(C+P+CONCAT+CARRYIN)
      | PCIN>>17-(C+P+P)
      | PCIN>>17-(C+P+P+CARRYIN)
      | PCIN>>17-(CARRYIN)
      | PCIN>>17-(CONCAT)
      | PCIN>>17-(CONCAT+C)
      | PCIN>>17-(CONCAT+C+C)
      | PCIN>>17-(CONCAT+C+C+CARRYIN)
      | PCIN>>17-(CONCAT+C+CARRYIN)
      | PCIN>>17-(CONCAT+C+P)
      | PCIN>>17-(CONCAT+C+P+CARRYIN)
      | PCIN>>17-(CONCAT+CARRYIN)
      | PCIN>>17-(CONCAT+P)
      | PCIN>>17-(CONCAT+P+C)
      | PCIN>>17-(CONCAT+P+C+CARRYIN)
      | PCIN>>17-(CONCAT+P+CARRYIN)
      | PCIN>>17-(D)
      | PCIN>>17-(D*B)
      | PCIN>>17-(D*B+C)
      | PCIN>>17-(D*B+C+CARRYIN)
      | PCIN>>17-(D*B+CARRYIN)
      | PCIN>>17-(D*B+P)
      | PCIN>>17-(D*B+P+CARRYIN)
      | PCIN>>17-(D*BCIN)
      | PCIN>>17-(D*BCIN+C)
      | PCIN>>17-(D*BCIN+C+CARRYIN)
      | PCIN>>17-(D*BCIN+CARRYIN)
      | PCIN>>17-(D*BCIN+P)
      | PCIN>>17-(D*BCIN+P+CARRYIN)
      | PCIN>>17-(D*D)
      | PCIN>>17-(D*D+C)
      | PCIN>>17-(D*D+C+CARRYIN)
      | PCIN>>17-(D*D+CARRYIN)
      | PCIN>>17-(D*D+P)
      | PCIN>>17-(D*D+P+CARRYIN)
      | PCIN>>17-(D+A)
      | PCIN>>17-(D+A)*(D+A)
      | PCIN>>17-(D+A)*(D+A)-C
      | PCIN>>17-(D+A)*(D+A)-C-CARRYIN
      | PCIN>>17-(D+A)*(D+A)-CARRYIN
      | PCIN>>17-(D+A)*(D+A)-P
      | PCIN>>17-(D+A)*(D+A)-P-CARRYIN
      | PCIN>>17-(D+A)*B
      | PCIN>>17-(D+A)*B-C
      | PCIN>>17-(D+A)*B-C-CARRYIN
      | PCIN>>17-(D+A)*B-CARRYIN
      | PCIN>>17-(D+A)*B-P
      | PCIN>>17-(D+A)*B-P-CARRYIN
      | PCIN>>17-(D+A)*BCIN
      | PCIN>>17-(D+A)*BCIN-C
      | PCIN>>17-(D+A)*BCIN-C-CARRYIN
      | PCIN>>17-(D+A)*BCIN-CARRYIN
      | PCIN>>17-(D+A)*BCIN-P
      | PCIN>>17-(D+A)*BCIN-P-CARRYIN
      | PCIN>>17-(D+A)-C
      | PCIN>>17-(D+A)-C-CARRYIN
      | PCIN>>17-(D+A)-CARRYIN
      | PCIN>>17-(D+A)-P
      | PCIN>>17-(D+A)-P-CARRYIN
      | PCIN>>17-(D+ACIN)
      | PCIN>>17-(D+ACIN)*(D+ACIN)
      | PCIN>>17-(D+ACIN)*(D+ACIN)-C
      | PCIN>>17-(D+ACIN)*(D+ACIN)-C-CARRYIN
      | PCIN>>17-(D+ACIN)*(D+ACIN)-CARRYIN
      | PCIN>>17-(D+ACIN)*(D+ACIN)-P
      | PCIN>>17-(D+ACIN)*(D+ACIN)-P-CARRYIN
      | PCIN>>17-(D+ACIN)*B
      | PCIN>>17-(D+ACIN)*B-C
      | PCIN>>17-(D+ACIN)*B-C-CARRYIN
      | PCIN>>17-(D+ACIN)*B-CARRYIN
      | PCIN>>17-(D+ACIN)*B-P
      | PCIN>>17-(D+ACIN)*B-P-CARRYIN
      | PCIN>>17-(D+ACIN)*BCIN
      | PCIN>>17-(D+ACIN)*BCIN-C
      | PCIN>>17-(D+ACIN)*BCIN-C-CARRYIN
      | PCIN>>17-(D+ACIN)*BCIN-CARRYIN
      | PCIN>>17-(D+ACIN)*BCIN-P
      | PCIN>>17-(D+ACIN)*BCIN-P-CARRYIN
      | PCIN>>17-(D+ACIN)-C
      | PCIN>>17-(D+ACIN)-C-CARRYIN
      | PCIN>>17-(D+ACIN)-CARRYIN
      | PCIN>>17-(D+ACIN)-P
      | PCIN>>17-(D+ACIN)-P-CARRYIN
      | PCIN>>17-(D+C)
      | PCIN>>17-(D+C+CARRYIN)
      | PCIN>>17-(D+CARRYIN)
      | PCIN>>17-(D+P)
      | PCIN>>17-(D+P+CARRYIN)
      | PCIN>>17-(D-A)
      | PCIN>>17-(D-A)*(D-A)
      | PCIN>>17-(D-A)*(D-A)-C
      | PCIN>>17-(D-A)*(D-A)-C-CARRYIN
      | PCIN>>17-(D-A)*(D-A)-CARRYIN
      | PCIN>>17-(D-A)*(D-A)-P
      | PCIN>>17-(D-A)*(D-A)-P-CARRYIN
      | PCIN>>17-(D-A)*B
      | PCIN>>17-(D-A)*B-C
      | PCIN>>17-(D-A)*B-C-CARRYIN
      | PCIN>>17-(D-A)*B-CARRYIN
      | PCIN>>17-(D-A)*B-P